simulink library "libturbines"  (R2025b)
settings: Solver=ode45  StartTime=0.0  StopTime=10.0  RelTol=1e-3  EnableMultiTasking=on
undefined symbols (not defined in the file or in its folder): DiscIntNeverNeededParam, dt, faultOptions, hydroFlag, noiseFlag
libraries: simulink x57, $bdroot x3
(part 1 of 5)
settings Model: Creator=grunnet  LastModifiedBy=yerai
settings SolverCC: AlgebraicLoopSolver=TrustRegion
settings DataIOCC: LimitDataPoints=on  SaveFormat=Array  SignalLoggingSaveFormat=ModelDataLogs  ReturnWorkspaceOutputs=off
settings OptimizationCC: ExpressionDepthLimit=2147483647.0  LifeSpan=inf
settings DebuggingCC: UnderspecifiedInitializationDetection=Classic  MergeDetectMultiDrivingBlocksExec=none  SolverPrmCheckMsg=warning  FcnCallInpInsideContextMsg=warning  UnconnectedInputMsg=warning  UnconnectedOutputMsg=warning  UnconnectedLineMsg=warning  StateNameClashWarn=warning  SFUnexpectedBacktrackingDiag=warning  SFNoUnconditionalDefaultTransitionDiag=warning  SFExecutionAtInitializationDiag=none  ArithmeticOperatorsInVariantConditions=warning
settings HardwareCC: ProdBitPerPointer=32.0  ProdBitPerSizeT=32.0  ProdBitPerPtrDiffT=32.0  ProdLargestAtomicFloat=None  ProdIntDivRoundTo=Undefined  ProdEndianess=Unspecified  ProdWordSize=32.0  ProdHWDeviceType=32-bit Generic
settings ModelReferenceCC: EnableRefExpFcnMdlSchedulingChecks=on  PropagateSignalLabelsOutOfModel=off
settings SFSimCC: SimUseLocalCustomCode=off  CustomCodeUndefinedFunction=UseInterfaceOnly
settings RTWCC: RTWUseLocalCustomCode=off  GPUComputeCapability=3.5  DLArmComputeVersion=19.05
settings CodeAppCC: StateflowObjectComments=on
settings GRTTargetCC: TargetLangStandard=C89/C90 (ANSI)  CombineOutputUpdateFcns=off  GRTInterface=on
settings ConfigComp: CovMetricSettings=dw  CovSaveCumulativeToWorkspaceVar=on  CovSaveSingleToWorkspaceVar=on  CovExternalEMLEnable=off

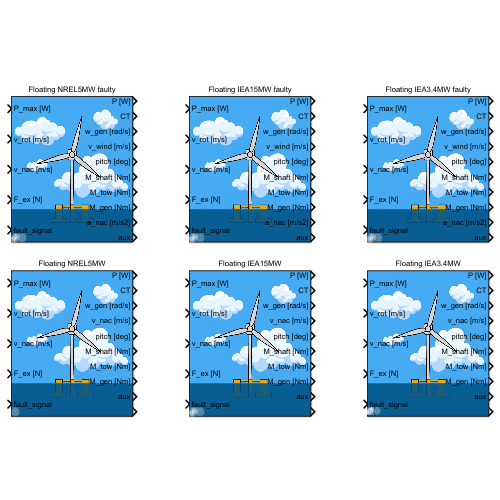
[thumbnail: Simulink's stored preview of the top-level diagram]
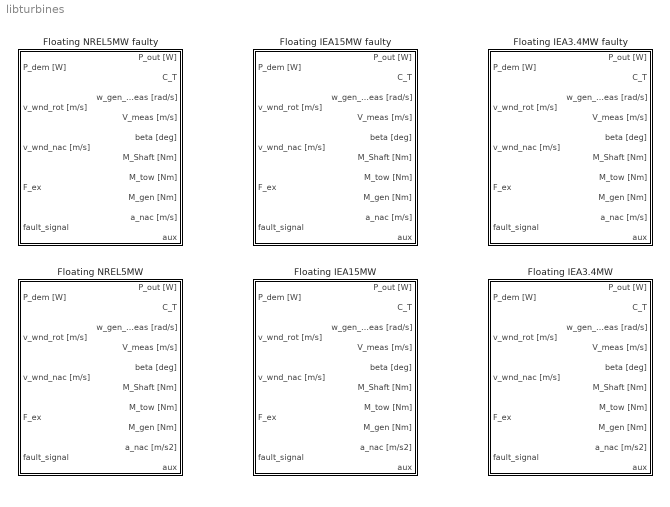
== system libturbines ==
  Floating IEA15MW : Subsystem  AncestorBlock=libturbines/Floating NREL5MW  mask(Wind Turbine){w_gen_ini=0.78788, beta_ini=0, auto=on, torq_ini=0}
      mask prompts: w_gen_ini "Initial generator velocity  [rad/s]"; beta_ini "Initial blade pitch [degrees]"; auto "Auto initial torque"; torq_ini "Initial generator torque"
      mask help: NREL 5MW Virtual Turbine
      mask init:
      | % l = load(file);
      | l               = get_param(gcb,'UserData');
      | env             = l.env;
      | wt              = l.wt;
      | plat            = l.plat;
      | wt.ctrl.gen.ini = w_gen_ini;
      | 
      | 
      | if(auto)
      |     wt.ctrl.torq.ini = min(interp1(wt.ctrl.torq.wg,wt.ctrl.torq.M,w_gen_ini,'spline'),wt.ctrl.torq.lim);
      | else
      |     wt.ctrl.torq.ini = torq_ini;
      | end
      | 
      | wt.pitch.ini        = beta_ini;
      | wt.ctrl.pitch.ini   = beta_ini;
      | wt.gen.torq.ini     = wt.ctrl.torq.ini;
      | wt.gen.ini          = w_gen_ini;
      | wt.rotor.ini        = w_gen_ini/wt.gen.N;
      mask Display:
      | image('FOWT.png');
      | 
      | port_label('output',1,'P [W]')
      | port_label('output',2,'CT')
      | port_label('output',3,'w_gen [rad/s]')
      | port_label('output',4,'v_nac [m/s]')
      | port_label('output',5,'pitch [deg]')
      | port_label('output',6,'M_shaft [Nm]')
      | port_label('output',7,'M_tow [Nm]')
      | port_label('output',8,'M_gen [Nm]')
      | port_label('output',9,'aux')
      | 
      | port_label('input',1,'P_max [W]')
      | port_label('input',2,'v_rot [m/s]')
      | port_label('input',3,'v_nac [m/s]')
      | port_label('input',4,'F_ex [N]')
      | port_label('input',5,'fault_signal')
  Floating IEA15MW faulty : Subsystem  AncestorBlock=libturbines/Floating NREL5MW faulty  mask(Wind Turbine){w_gen_ini=0.78788, beta_ini=0, auto=on, torq_ini=0}
      mask prompts: w_gen_ini "Initial generator velocity  [rad/s]"; beta_ini "Initial blade pitch [degrees]"; auto "Auto initial torque"; torq_ini "Initial generator torque"
      mask help: NREL 5MW Virtual Turbine
      mask init: same as libturbines/Floating IEA15MW
      mask Display:
      | image('FOWT.png');
      | 
      | port_label('output',1,'P [W]')
      | port_label('output',2,'CT')
      | port_label('output',3,'w_gen [rad/s]')
      | port_label('output',4,'v_wind [m/s]')
      | port_label('output',5,'pitch [deg]')
      | port_label('output',6,'M_shaft [Nm]')
      | port_label('output',7,'M_tow [Nm]')
      | port_label('output',8,'M_gen [Nm]')
      | port_label('output',9,'a_nac [m/s2]')
      | port_label('output',10,'aux')
      | 
      | port_label('input',1,'P_max [W]')
      | port_label('input',2,'v_rot [m/s]')
      | port_label('input',3,'v_nac [m/s]')
      | port_label('input',4,'F_ex [N]')
      | port_label('input',5,'fault_signal')
  Floating IEA3.4MW : Subsystem  AncestorBlock=libturbines/Floating IEA15MW  mask(Wind Turbine){w_gen_ini=118.1754, beta_ini=0, auto=on, torq_ini=0}
      mask prompts: w_gen_ini "Initial generator velocity  [rad/s]"; beta_ini "Initial blade pitch [degrees]"; auto "Auto initial torque"; torq_ini "Initial generator torque"
      mask help: NREL 5MW Virtual Turbine
      mask init: same as libturbines/Floating IEA15MW
      mask Display:
      | image('FOWT.png');
      | 
      | port_label('output',1,'P [W]')
      | port_label('output',2,'CT')
      | port_label('output',3,'w_gen [rad/s]')
      | port_label('output',4,'v_nac [m/s]')
      | port_label('output',5,'pitch [deg]')
      | port_label('output',6,'M_shaft [Nm]')
      | port_label('output',7,'M_tow [Nm]')
      | port_label('output',8,'M_gen [Nm]')
      | port_label('output',9,'aux')
      | 
      | port_label('input',1,'P_max [W]')
      | port_label('input',2,'v_rot [m/s]')
      | port_label('input',3,'v_nac [m/s]')
      | port_label('input',4,'F_ex [N]')
      | port_label('input',5,'fault_signal')
  Floating IEA3.4MW faulty : Subsystem  AncestorBlock=libturbines/Floating IEA15MW faulty  mask(Wind Turbine){w_gen_ini=118.1754, beta_ini=0, auto=on, torq_ini=0}
      mask prompts: w_gen_ini "Initial generator velocity  [rad/s]"; beta_ini "Initial blade pitch [degrees]"; auto "Auto initial torque"; torq_ini "Initial generator torque"
      mask help: NREL 5MW Virtual Turbine
      mask init: same as libturbines/Floating IEA15MW
      mask Display:
      | image('FOWT.png');
      | 
      | port_label('output',1,'P [W]')
      | port_label('output',2,'CT')
      | port_label('output',3,'w_gen [rad/s]')
      | port_label('output',4,'v_wind [m/s]')
      | port_label('output',5,'pitch [deg]')
      | port_label('output',6,'M_shaft [Nm]')
      | port_label('output',7,'M_tow [Nm]')
      | port_label('output',8,'M_gen [Nm]')
      | port_label('output',9,'a_nac [m/s2]')
      | port_label('output',10,'aux')
      | 
      | port_label('input',1,'P_max [W]')
      | port_label('input',2,'v_rot [m/s]')
      | port_label('input',3,'v_nac [m/s]')
      | port_label('input',4,'F_ex [N]')
      | port_label('input',5,'fault_signal')
  Floating NREL5MW : Subsystem  mask(Wind Turbine){w_gen_ini=50, beta_ini=0, auto=off, torq_ini=0}
      mask prompts: w_gen_ini "Initial generator velocity  [rad/s]"; beta_ini "Initial blade pitch [degrees]"; auto "Auto initial torque"; torq_ini "Initial generator torque"
      mask help: NREL 5MW Virtual Turbine
      mask init: same as libturbines/Floating IEA15MW
      mask Display:
      | image('FOWT.png');
      | 
      | port_label('output',1,'P [W]')
      | port_label('output',2,'CT')
      | port_label('output',3,'w_gen [rad/s]')
      | port_label('output',4,'v_nac [m/s]')
      | port_label('output',5,'pitch [deg]')
      | port_label('output',6,'M_shaft [Nm]')
      | port_label('output',7,'M_tow [Nm]')
      | port_label('output',8,'M_gen [Nm]')
      | port_label('output',9,'aux')
      | 
      | port_label('input',1,'P_max [W]')
      | port_label('input',2,'v_rot [m/s]')
      | port_label('input',3,'v_nac [m/s]')
      | port_label('input',4,'F_ex [N]')
      | port_label('input',5,'fault_signal')
  Floating NREL5MW faulty : Subsystem  AncestorBlock=libturbines/Faulty NREL5MW  mask(Wind Turbine){w_gen_ini=50, beta_ini=0, auto=off, torq_ini=0}
      mask prompts: w_gen_ini "Initial generator velocity  [rad/s]"; beta_ini "Initial blade pitch [degrees]"; auto "Auto initial torque"; torq_ini "Initial generator torque"
      mask help: NREL 5MW Virtual Turbine
      mask init: same as libturbines/Floating IEA15MW
      mask Display:
      | image('FOWT.png');
      | 
      | port_label('output',1,'P [W]')
      | port_label('output',2,'CT')
      | port_label('output',3,'w_gen [rad/s]')
      | port_label('output',4,'v_wind [m/s]')
      | port_label('output',5,'pitch [deg]')
      | port_label('output',6,'M_shaft [Nm]')
      | port_label('output',7,'M_tow [Nm]')
      | port_label('output',8,'M_gen [Nm]')
      | port_label('output',9,'a_nac [m/s2]')
      | port_label('output',10,'aux')
      | 
      | port_label('input',1,'P_max [W]')
      | port_label('input',2,'v_rot [m/s]')
      | port_label('input',3,'v_nac [m/s]')
      | port_label('input',4,'F_ex [N]')
      | port_label('input',5,'fault_signal')

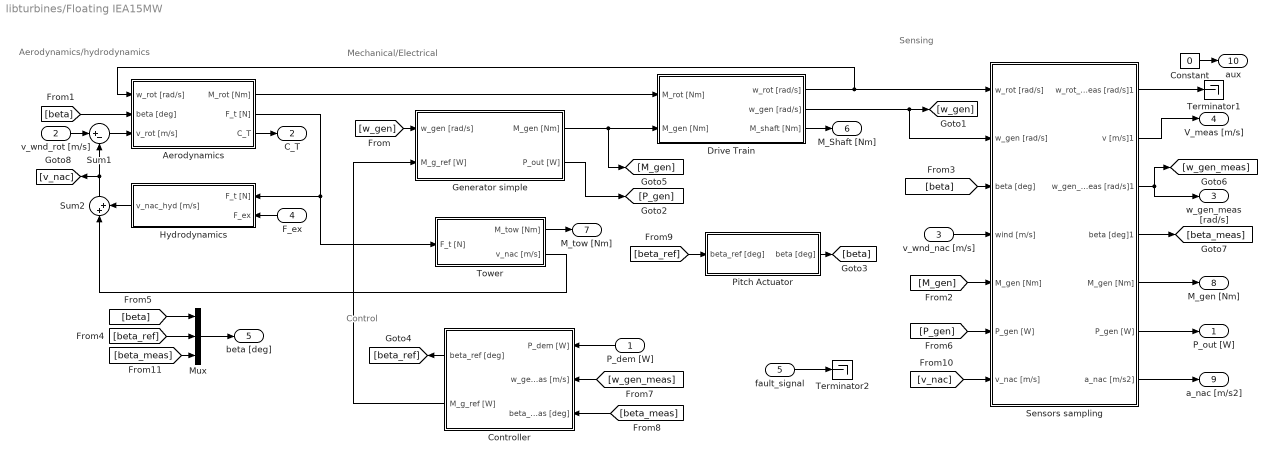
== system libturbines/Floating IEA15MW ==
  note: Control
  note: Aerodynamics/hydrodynamics
  note: Mechanical/Electrical
  note: Sensing
  P_dem [W] : Inport
  v_wnd_rot [m/s] : Inport  Port=2
  v_wnd_nac [m/s] : Inport  Port=3
  F_ex : Inport  Port=4
  fault_signal : Inport  Port=5
  Aerodynamics : Subsystem
  Constant : Constant  Value=0
  Controller : Subsystem
  Drive Train : Subsystem
  Generator simple : Subsystem
  Hydrodynamics : Subsystem
  Mux : Mux  Inputs=3
  Pitch Actuator : Subsystem
  Sensors sampling : Subsystem
  Sum1 : Sum  Inputs=|+-  InputSameDT=on  SaturateOnIntegerOverflow=on  OutDataTypeStr=Inherit: Same as first input
  Sum2 : Sum  Inputs=|++  InputSameDT=on  SaturateOnIntegerOverflow=on  OutDataTypeStr=Inherit: Same as first input
  Terminator1 : Terminator
  Terminator2 : Terminator
  "Tower " : Subsystem
  P_out [W] : Outport
  C_T : Outport  Port=2
  w_gen_meas [rad/s] : Outport  Port=3
  V_meas [m/s] : Outport  Port=4
  beta [deg] : Outport  Port=5
  M_Shaft [Nm] : Outport  Port=6
  M_tow [Nm] : Outport  Port=7
  M_gen [Nm] : Outport  Port=8
  a_nac [m/s2] : Outport  Port=9
  aux : Outport  Port=10
  wires:
  Sensors sampling/7(a_nac [m/s2]) -> a_nac [m/s2]/1
  [v_nac] -> Sensors sampling/7("v_nac [m/s] ")
  "Tower "/2(v_nac [m/s]) -> Sum2/1
  Sum2/1 -> Sum1/2, [v_nac]
  Hydrodynamics/1(v_nac_hyd [m/s]) -> Sum2/2
  [P_gen] -> Sensors sampling/6("P_gen [W] ")
  [M_gen] -> Sensors sampling/5("M_gen [Nm] ")
  Generator simple/2(P_out [W]) -> [P_gen]
  Sensors sampling/6(P_gen [W]) -> P_out [W]/1
  Sensors sampling/5(M_gen [Nm]) -> M_gen [Nm]/1
  Sensors sampling/4(beta [deg]1) -> [beta_meas]
  Sensors sampling/3(w_gen_meas [rad/s]1) -> [w_gen_meas], w_gen_meas [rad/s]/1
  Sensors sampling/2(v [m/s]1) -> V_meas [m/s]/1
  Sensors sampling/1(w_rot_meas [rad/s]1) -> Terminator1/1
  [beta] -> Aerodynamics/2(beta [deg])
  [w_gen] -> Generator simple/1(w_gen [rad/s])
  fault_signal/1 -> Terminator2/1
  Drive Train/3(M_shaft [Nm]) -> M_Shaft [Nm]/1
  v_wnd_nac [m/s]/1 -> Sensors sampling/4(wind [m/s])
  v_wnd_rot [m/s]/1 -> Sum1/1
  "Tower "/1(M_tow [Nm]) -> M_tow [Nm]/1
  Aerodynamics/2(F_t [N]) -> Hydrodynamics/1(F_t [N]), "Tower "/1(F_t [N])
  Pitch Actuator/1(beta [deg]) -> [beta]
  Controller/1(beta_ref [deg]) -> [beta_ref]
  P_dem [W]/1 -> Controller/1(P_dem [W])
  Controller/2(M_g_ref [W]) -> Generator simple/2(M_g_ref [W])
  Sum1/1 -> Aerodynamics/3(v_rot [m/s])
  Drive Train/2(w_gen [rad/s]) -> [w_gen], Sensors sampling/2(w_gen [rad/s])
  Generator simple/1(M_gen [Nm]) -> Drive Train/2(M_gen [Nm]), [M_gen]
  Drive Train/1(w_rot [rad/s]) -> Aerodynamics/1(w_rot [rad/s]), Sensors sampling/1("w_rot [rad/s] ")
  Aerodynamics/1(M_rot [Nm]) -> Drive Train/1(M_rot [Nm])
  Aerodynamics/3(C_T) -> C_T/1
  [beta] -> Sensors sampling/3("beta [deg] ")
  [beta_ref] -> Mux/2
  [beta] -> Mux/1
  [w_gen_meas] -> Controller/2(w_gen_meas [m/s])
  [beta_meas] -> Controller/3(beta_meas [deg])
  [beta_ref] -> Pitch Actuator/1(beta_ref [deg])
  [beta_meas] -> Mux/3
  F_ex/1 -> Hydrodynamics/2(F_ex)
  Mux/1 -> beta [deg]/1
  Constant/1 -> aux/1

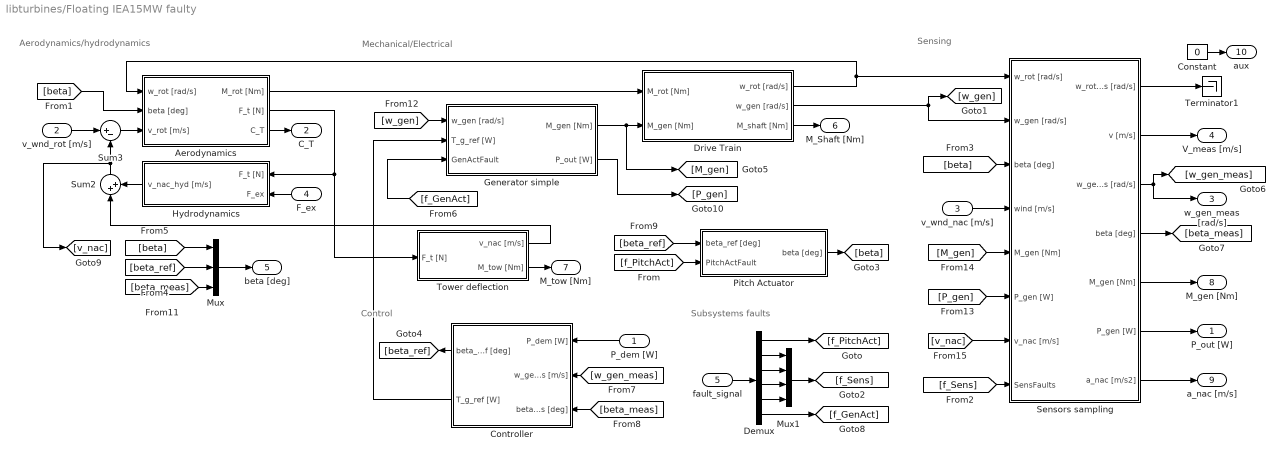
== system libturbines/Floating IEA15MW faulty ==
  note: Subsystems faults
  note: Sensing
  note: Mechanical/Electrical
  note: Aerodynamics/hydrodynamics
  note: Control
  P_dem [W] : Inport
  v_wnd_rot [m/s] : Inport  Port=2
  v_wnd_nac [m/s] : Inport  Port=3
  F_ex : Inport  Port=4
  fault_signal : Inport  Port=5
  Aerodynamics : Subsystem
  Constant : Constant  Value=0
  Controller : Subsystem
  Demux : Demux  Outputs=6
  Drive Train : Subsystem
  Generator simple : Subsystem
  Hydrodynamics : Subsystem
  Mux : Mux  Inputs=3
  Mux1 : Mux
  Pitch Actuator : Subsystem
  Sensors sampling : Subsystem
  Sum2 : Sum  Inputs=|++  InputSameDT=on  SaturateOnIntegerOverflow=on  OutDataTypeStr=Inherit: Same as first input
  Sum3 : Sum  Inputs=|+-  InputSameDT=on  SaturateOnIntegerOverflow=on  OutDataTypeStr=Inherit: Same as first input
  Terminator1 : Terminator
  "Tower deflection " : Subsystem
  P_out [W] : Outport
  C_T : Outport  Port=2
  w_gen_meas [rad/s] : Outport  Port=3
  V_meas [m/s] : Outport  Port=4
  beta [deg] : Outport  Port=5
  M_Shaft [Nm] : Outport  Port=6
  M_tow [Nm] : Outport  Port=7
  M_gen [Nm] : Outport  Port=8
  a_nac [m/s] : Outport  Port=9
  aux : Outport  Port=10
  wires:
  Demux/5 -> Mux1/4
  Demux/6 -> [f_GenAct]
  Sensors sampling/7(a_nac [m/s2]) -> a_nac [m/s]/1
  [v_nac] -> Sensors sampling/7("v_nac [m/s] ")
  "Tower deflection "/1(v_nac [m/s]) -> Sum2/1
  Sum2/1 -> Sum3/2, [v_nac]
  Hydrodynamics/1(v_nac_hyd [m/s]) -> Sum2/2
  v_wnd_rot [m/s]/1 -> Sum3/1
  Sum3/1 -> Aerodynamics/3(v_rot [m/s])
  [M_gen] -> Sensors sampling/5("M_gen [Nm] ")
  [P_gen] -> Sensors sampling/6("P_gen [W] ")
  Generator simple/2(P_out [W]) -> [P_gen]
  Sensors sampling/5(M_gen [Nm]) -> M_gen [Nm]/1
  Sensors sampling/6(P_gen [W]) -> P_out [W]/1
  [w_gen] -> Generator simple/1(w_gen [rad/s])
  [beta] -> Aerodynamics/2(beta [deg])
  Mux1/1 -> [f_Sens]
  Demux/4 -> Mux1/3
  Demux/2 -> Mux1/1
  Demux/3 -> Mux1/2
  Constant/1 -> aux/1
  Mux/1 -> beta [deg]/1
  F_ex/1 -> Hydrodynamics/2(F_ex)
  [beta_meas] -> Mux/3
  [beta_ref] -> Pitch Actuator/1(beta_ref [deg])
  [beta_meas] -> Controller/3(beta_meas [deg])
  [w_gen_meas] -> Controller/2(w_gen_meas [m/s])
  [f_GenAct] -> Generator simple/3(GenActFault)
  [beta] -> Mux/1
  [beta_ref] -> Mux/2
  [beta] -> Sensors sampling/3("beta [deg] ")
  [f_Sens] -> Sensors sampling/8(SensFaults)
  [f_PitchAct] -> Pitch Actuator/2(PitchActFault)
  Demux/1 -> [f_PitchAct]
  fault_signal/1 -> Demux/1
  Aerodynamics/3(C_T) -> C_T/1
  Aerodynamics/1(M_rot [Nm]) -> Drive Train/1(M_rot [Nm])
  Drive Train/1(w_rot [rad/s]) -> Sensors sampling/1("w_rot [rad/s] "), Aerodynamics/1(w_rot [rad/s])
  Generator simple/1(M_gen [Nm]) -> Drive Train/2(M_gen [Nm]), [M_gen]
  Drive Train/2(w_gen [rad/s]) -> Sensors sampling/2(w_gen [rad/s]), [w_gen]
  Sensors sampling/4(beta [deg]) -> [beta_meas]
  Sensors sampling/2(v [m/s]) -> V_meas [m/s]/1
  Controller/2(T_g_ref [W]) -> Generator simple/2(T_g_ref [W])
  Sensors sampling/3(w_gen_meas [rad/s]) -> [w_gen_meas], w_gen_meas [rad/s]/1
  P_dem [W]/1 -> Controller/1(P_dem [W])
  Controller/1(beta_ref [deg]) -> [beta_ref]
  Pitch Actuator/1(beta [deg]) -> [beta]
  Aerodynamics/2(F_t [N]) -> Hydrodynamics/1(F_t [N]), "Tower deflection "/1(F_t [N])
  "Tower deflection "/2(M_tow [Nm]) -> M_tow [Nm]/1
  v_wnd_nac [m/s]/1 -> Sensors sampling/4(wind [m/s])
  Drive Train/3(M_shaft [Nm]) -> M_Shaft [Nm]/1
  Sensors sampling/1(w_rot_meas [rad/s]) -> Terminator1/1

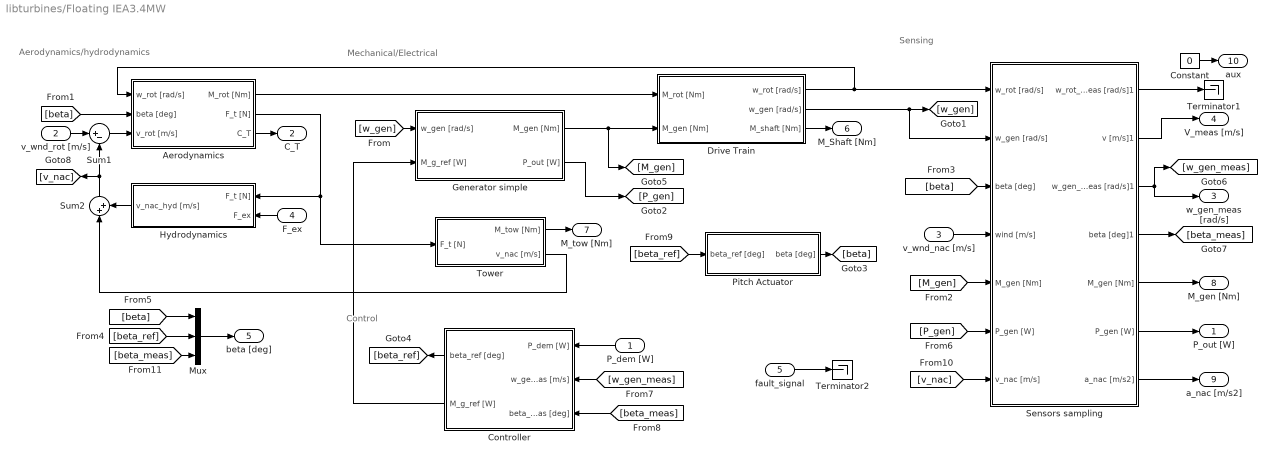
== system libturbines/Floating IEA3.4MW ==
  note: Control
  note: Aerodynamics/hydrodynamics
  note: Mechanical/Electrical
  note: Sensing
  P_dem [W] : Inport
  v_wnd_rot [m/s] : Inport  Port=2
  v_wnd_nac [m/s] : Inport  Port=3
  F_ex : Inport  Port=4
  fault_signal : Inport  Port=5
  Aerodynamics : Subsystem
  Constant : Constant  Value=0
  Controller : Subsystem
  Drive Train : Subsystem
  Generator simple : Subsystem
  Hydrodynamics : Subsystem
  Mux : Mux  Inputs=3
  Pitch Actuator : Subsystem
  Sensors sampling : Subsystem
  Sum1 : Sum  Inputs=|+-  InputSameDT=on  SaturateOnIntegerOverflow=on  OutDataTypeStr=Inherit: Same as first input
  Sum2 : Sum  Inputs=|++  InputSameDT=on  SaturateOnIntegerOverflow=on  OutDataTypeStr=Inherit: Same as first input
  Terminator1 : Terminator
  Terminator2 : Terminator
  "Tower " : Subsystem
  P_out [W] : Outport
  C_T : Outport  Port=2
  w_gen_meas [rad/s] : Outport  Port=3
  V_meas [m/s] : Outport  Port=4
  beta [deg] : Outport  Port=5
  M_Shaft [Nm] : Outport  Port=6
  M_tow [Nm] : Outport  Port=7
  M_gen [Nm] : Outport  Port=8
  a_nac [m/s2] : Outport  Port=9
  aux : Outport  Port=10
  wires:
  Sensors sampling/7(a_nac [m/s2]) -> a_nac [m/s2]/1
  [v_nac] -> Sensors sampling/7("v_nac [m/s] ")
  "Tower "/2(v_nac [m/s]) -> Sum2/1
  Sum2/1 -> Sum1/2, [v_nac]
  Hydrodynamics/1(v_nac_hyd [m/s]) -> Sum2/2
  [P_gen] -> Sensors sampling/6("P_gen [W] ")
  [M_gen] -> Sensors sampling/5("M_gen [Nm] ")
  Generator simple/2(P_out [W]) -> [P_gen]
  Sensors sampling/6(P_gen [W]) -> P_out [W]/1
  Sensors sampling/5(M_gen [Nm]) -> M_gen [Nm]/1
  Sensors sampling/4(beta [deg]1) -> [beta_meas]
  Sensors sampling/3(w_gen_meas [rad/s]1) -> [w_gen_meas], w_gen_meas [rad/s]/1
  Sensors sampling/2(v [m/s]1) -> V_meas [m/s]/1
  Sensors sampling/1(w_rot_meas [rad/s]1) -> Terminator1/1
  [beta] -> Aerodynamics/2(beta [deg])
  [w_gen] -> Generator simple/1(w_gen [rad/s])
  fault_signal/1 -> Terminator2/1
  Drive Train/3(M_shaft [Nm]) -> M_Shaft [Nm]/1
  v_wnd_nac [m/s]/1 -> Sensors sampling/4(wind [m/s])
  v_wnd_rot [m/s]/1 -> Sum1/1
  "Tower "/1(M_tow [Nm]) -> M_tow [Nm]/1
  Aerodynamics/2(F_t [N]) -> Hydrodynamics/1(F_t [N]), "Tower "/1(F_t [N])
  Pitch Actuator/1(beta [deg]) -> [beta]
  Controller/1(beta_ref [deg]) -> [beta_ref]
  P_dem [W]/1 -> Controller/1(P_dem [W])
  Controller/2(M_g_ref [W]) -> Generator simple/2(M_g_ref [W])
  Sum1/1 -> Aerodynamics/3(v_rot [m/s])
  Drive Train/2(w_gen [rad/s]) -> [w_gen], Sensors sampling/2(w_gen [rad/s])
  Generator simple/1(M_gen [Nm]) -> Drive Train/2(M_gen [Nm]), [M_gen]
  Drive Train/1(w_rot [rad/s]) -> Aerodynamics/1(w_rot [rad/s]), Sensors sampling/1("w_rot [rad/s] ")
  Aerodynamics/1(M_rot [Nm]) -> Drive Train/1(M_rot [Nm])
  Aerodynamics/3(C_T) -> C_T/1
  [beta] -> Sensors sampling/3("beta [deg] ")
  [beta_ref] -> Mux/2
  [beta] -> Mux/1
  [w_gen_meas] -> Controller/2(w_gen_meas [m/s])
  [beta_meas] -> Controller/3(beta_meas [deg])
  [beta_ref] -> Pitch Actuator/1(beta_ref [deg])
  [beta_meas] -> Mux/3
  F_ex/1 -> Hydrodynamics/2(F_ex)
  Mux/1 -> beta [deg]/1
  Constant/1 -> aux/1

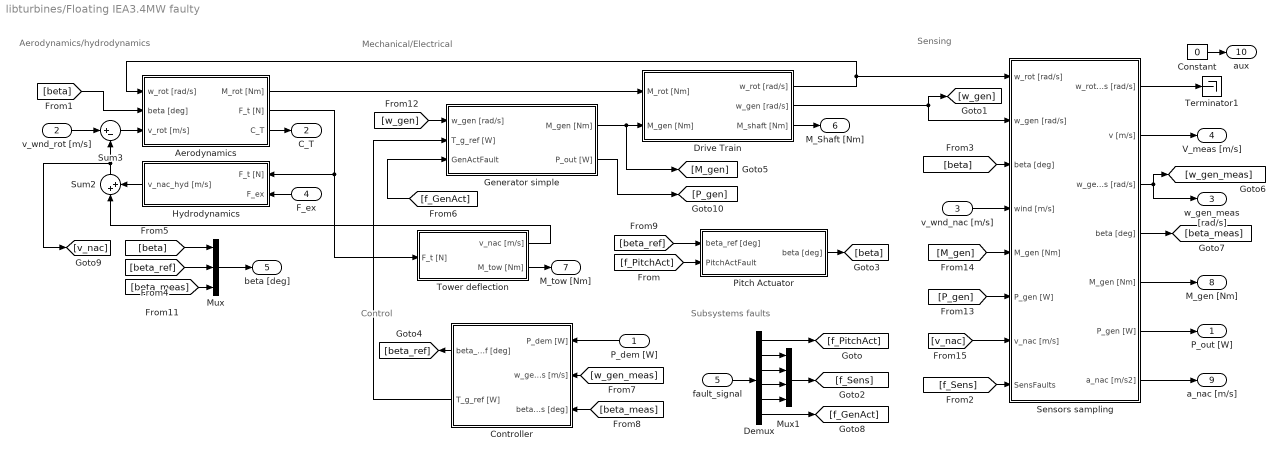
== system libturbines/Floating IEA3.4MW faulty ==
  note: Subsystems faults
  note: Sensing
  note: Mechanical/Electrical
  note: Aerodynamics/hydrodynamics
  note: Control
  P_dem [W] : Inport
  v_wnd_rot [m/s] : Inport  Port=2
  v_wnd_nac [m/s] : Inport  Port=3
  F_ex : Inport  Port=4
  fault_signal : Inport  Port=5
  Aerodynamics : Subsystem
  Constant : Constant  Value=0
  Controller : Subsystem
  Demux : Demux  Outputs=6
  Drive Train : Subsystem
  Generator simple : Subsystem
  Hydrodynamics : Subsystem
  Mux : Mux  Inputs=3
  Mux1 : Mux
  Pitch Actuator : Subsystem
  Sensors sampling : Subsystem
  Sum2 : Sum  Inputs=|++  InputSameDT=on  SaturateOnIntegerOverflow=on  OutDataTypeStr=Inherit: Same as first input
  Sum3 : Sum  Inputs=|+-  InputSameDT=on  SaturateOnIntegerOverflow=on  OutDataTypeStr=Inherit: Same as first input
  Terminator1 : Terminator
  "Tower deflection " : Subsystem
  P_out [W] : Outport
  C_T : Outport  Port=2
  w_gen_meas [rad/s] : Outport  Port=3
  V_meas [m/s] : Outport  Port=4
  beta [deg] : Outport  Port=5
  M_Shaft [Nm] : Outport  Port=6
  M_tow [Nm] : Outport  Port=7
  M_gen [Nm] : Outport  Port=8
  a_nac [m/s] : Outport  Port=9
  aux : Outport  Port=10
  wires:
  Demux/5 -> Mux1/4
  Demux/6 -> [f_GenAct]
  Sensors sampling/7(a_nac [m/s2]) -> a_nac [m/s]/1
  [v_nac] -> Sensors sampling/7("v_nac [m/s] ")
  "Tower deflection "/1(v_nac [m/s]) -> Sum2/1
  Sum2/1 -> Sum3/2, [v_nac]
  Hydrodynamics/1(v_nac_hyd [m/s]) -> Sum2/2
  v_wnd_rot [m/s]/1 -> Sum3/1
  Sum3/1 -> Aerodynamics/3(v_rot [m/s])
  [M_gen] -> Sensors sampling/5("M_gen [Nm] ")
  [P_gen] -> Sensors sampling/6("P_gen [W] ")
  Generator simple/2(P_out [W]) -> [P_gen]
  Sensors sampling/5(M_gen [Nm]) -> M_gen [Nm]/1
  Sensors sampling/6(P_gen [W]) -> P_out [W]/1
  [w_gen] -> Generator simple/1(w_gen [rad/s])
  [beta] -> Aerodynamics/2(beta [deg])
  Mux1/1 -> [f_Sens]
  Demux/4 -> Mux1/3
  Demux/2 -> Mux1/1
  Demux/3 -> Mux1/2
  Constant/1 -> aux/1
  Mux/1 -> beta [deg]/1
  F_ex/1 -> Hydrodynamics/2(F_ex)
  [beta_meas] -> Mux/3
  [beta_ref] -> Pitch Actuator/1(beta_ref [deg])
  [beta_meas] -> Controller/3(beta_meas [deg])
  [w_gen_meas] -> Controller/2(w_gen_meas [m/s])
  [f_GenAct] -> Generator simple/3(GenActFault)
  [beta] -> Mux/1
  [beta_ref] -> Mux/2
  [beta] -> Sensors sampling/3("beta [deg] ")
  [f_Sens] -> Sensors sampling/8(SensFaults)
  [f_PitchAct] -> Pitch Actuator/2(PitchActFault)
  Demux/1 -> [f_PitchAct]
  fault_signal/1 -> Demux/1
  Aerodynamics/3(C_T) -> C_T/1
  Aerodynamics/1(M_rot [Nm]) -> Drive Train/1(M_rot [Nm])
  Drive Train/1(w_rot [rad/s]) -> Sensors sampling/1("w_rot [rad/s] "), Aerodynamics/1(w_rot [rad/s])
  Generator simple/1(M_gen [Nm]) -> Drive Train/2(M_gen [Nm]), [M_gen]
  Drive Train/2(w_gen [rad/s]) -> Sensors sampling/2(w_gen [rad/s]), [w_gen]
  Sensors sampling/4(beta [deg]) -> [beta_meas]
  Sensors sampling/2(v [m/s]) -> V_meas [m/s]/1
  Controller/2(T_g_ref [W]) -> Generator simple/2(T_g_ref [W])
  Sensors sampling/3(w_gen_meas [rad/s]) -> [w_gen_meas], w_gen_meas [rad/s]/1